simulink model "AcasXuClosedLoop_m_1_comp2017b"  (Simulink 9.0)
settings: Solver=VariableStepAuto  StartTime=0.0  StopTime=35  MaxStep=0.2  RelTol=1e-3
libraries: neural x1560, simulink x3
(part 5 of 60)

== system AcasXuClosedLoop_m_1_comp2017b/networks/AcasXu_5_1/Layer 3 ==
  "a{2} " : Inport  PortDimensions=50
      mask Display: e = 0.06;c1 = [120 230 180]/255;c2 = ([120 230 180]+0)/(256+128);c3 = ([120 230 180]+128)/(256+128);patch([0 1 1 0],[0 0 1 1],c1);patch([0 1 1 1-e 1-e 0+e],[0 0 1 1-e 0+e 0+e],c2);patch([0 0 1 1-e 0+e 0+e],[0 1 1 1-e 1-e 0+e],c3);plot([0 1 1 0 0],[0 0 1 1 0]);
  Delays 1 : Subsystem
      mask Display: e = 0.06;c1 = [120 150 230]/255;c2 = ([120 150 230]+0)/383;c3 = ([120 150 230]+128)/383;patch([0 1 1 0],[0 0 1 1],c1);patch([0 1 1 1-e 1-e 0+e],[0 0 1 1-e 0+e 0+e],c2);patch([0 0 1 1-e 0+e 0+e],[0 1 1 1-e 1-e 0+e],c3);plot([0 1 1 0 0],[0 0 1 1 0]);disp('0');
  "LW{3,2}" : Subsystem
      mask Display: e = 0.06;c1 = [120 150 230]/255;c2 = ([120 150 230]+0)/383;c3 = ([120 150 230]+128)/383;patch([0 1 1 0],[0 0 1 1],c1);patch([0 1 1 1-e 1-e 0+e],[0 0 1 1-e 0+e 0+e],c2);patch([0 0 1 1-e 0+e 0+e],[0 1 1 1-e 1-e 0+e],c3);plot([0 1 1 0 0],[0 0 1 1 0]);disp('W');
  "b{3}" : Constant  Value=[0.272230000000000027515767442309879697859287261962890625;-1.0544800000000000839150970932678319513797760009765625;1.5729699999999999793232063893810845911502838134765625;1.4368700000000000915889586394769139587879180908203125;-0.422177999999999997715605104531277902424335479736328125;-0.98838400000000004030908939967048354446887969970703125;-0.021854800000000000614885919958396698348224163055419921875;-0.293798999999999976839859527899534441530704498291015625;1.0949400000000000243716158365714363753795623779296875;-0.0307898999999999985199838903326963190920650959014892578125;-0.06296770000000000144790845979514415375888347625732421875;-0.84011599999999997390176531553152017295360565185546875;0.503924000000000038568259697058238089084625244140625;0.2310029999999999861248767274446436204016208648681640625;-0.0287351999999999989376942011176652158610522747039794921875;1.1389400000000000634514663033769465982913970947265625;0.73577899999999996083488440490327775478363037109375;0.110875000000000001332267629550187848508358001708984375;-1.0117599999999999926814098216709680855274200439453125;-0.1491240000000000065494276668687234632670879364013671875;-1.0125100000000000211031192520749755203723907470703125;-1.1087100000000000843414227347238920629024505615234375;1.4243699999999999139532746994518674910068511962890625;0.298072999999999976861175809972337447106838226318359375;0.34876600000000002044231450781808234751224517822265625;0.277681000000000011151968237754772417247295379638671875;-1.259819999999999939888084554695524275302886962890625;-0.26194499999999998340882712000166065990924835205078125;0.14417299999999999560174046564497984945774078369140625;-0.29806100000000002037126023424207232892513275146484375;-1.0689100000000000267874611381557770073413848876953125;-0.0578330999999999983973708594930940307676792144775390625;0.600875999999999965694996717502363026142120361328125;0.61764699999999994606270092845079489052295684814453125;0.65339300000000000157029944602982141077518463134765625;-2.78967000000000009407585821463726460933685302734375;0.57990299999999994629007460389402695000171661376953125;0.480266000000000026215474235868896357715129852294921875;0.343100000000000016076029396572266705334186553955078125;-0.01002399999999999992417176741810180828906595706939697265625;-0.26819300000000001471534005759167484939098358154296875;-0.0729852000000000000756728013584506697952747344970703125;-0.0917779999999999984705567612763843499124050140380859375;0.446454999999999990745180866724695079028606414794921875;0.565514999999999989910293152206577360630035400390625;-0.54441200000000000702726765666739083826541900634765625;0.2388879999999999892423829805920831859111785888671875;0.0464861000000000024190427438952610827982425689697265625;0.55525100000000005007194658901426009833812713623046875;0.09514330000000000009396927680427324958145618438720703125]
      mask Display: e = 0.06;c1 = [120 150 230]/255;c2 = ([120 150 230]+0)/383;c3 = ([120 150 230]+128)/383;patch([0 1 1 0],[0 0 1 1],c1);patch([0 1 1 1-e 1-e 0+e],[0 0 1 1-e 0+e 0+e],c2);patch([0 0 1 1-e 0+e 0+e],[0 1 1 1-e 1-e 0+e],c3);plot([0 1 1 0 0],[0 0 1 1 0]);disp('b');
  netsum : Sum  Inputs=2  SaturateOnIntegerOverflow=on  InputSameDT=on  OutDataTypeStr=Inherit: Same as first input
      mask Display: e = 0.06;c1 = [120 150 230]/255;c2 = ([120 150 230]+0)/383;c3 = ([120 150 230]+128)/383;patch([0 1 1 0],[0 0 1 1],c1);patch([0 1 1 1-e 1-e 0+e],[0 0 1 1-e 0+e 0+e],c2);patch([0 0 1 1-e 0+e 0+e],[0 1 1 1-e 1-e 0+e],c3);plot([0 1 1 0 0],[0 0 1 1 0]);disp('+');
  poslin : neural/Transfer Functions/poslin
      settings: SourceProductName=Deep Learning Toolbox  SourceProductBaseCode=NN
  "a{3}" : Outport  InitialOutput=[0;0;0;0;0;0;0;0;0;0;0;0;0;0;0;0;0;0;0;0;0;0;0;0;0;0;0;0;0;0;0;0;0;0;0;0;0;0;0;0;0;0;0;0;0;0;0;0;0;0]
      mask Display: e = 0.06;c1 = [120 230 180]/255;c2 = ([120 230 180]+0)/(256+128);c3 = ([120 230 180]+128)/(256+128);patch([0 1 1 0],[0 0 1 1],c1);patch([0 1 1 1-e 1-e 0+e],[0 0 1 1-e 0+e 0+e],c2);patch([0 0 1 1-e 0+e 0+e],[0 1 1 1-e 1-e 0+e],c3);plot([0 1 1 0 0],[0 0 1 1 0]);
  wires:
  poslin/1 -> "a{3}"/1
  netsum/1 -> poslin/1
  "b{3}"/1 -> netsum/2
  "LW{3,2}"/1("lz{3,2}") -> netsum/1
  Delays 1/1("pd{3,2}") -> "LW{3,2}"/1("ad{3,2}")
  "a{2} "/1 -> Delays 1/1("p{3}")

== system AcasXuClosedLoop_m_1_comp2017b/networks/AcasXu_5_1/Layer 4 ==
  "a{3} " : Inport  PortDimensions=50
      mask Display: e = 0.06;c1 = [120 230 180]/255;c2 = ([120 230 180]+0)/(256+128);c3 = ([120 230 180]+128)/(256+128);patch([0 1 1 0],[0 0 1 1],c1);patch([0 1 1 1-e 1-e 0+e],[0 0 1 1-e 0+e 0+e],c2);patch([0 0 1 1-e 0+e 0+e],[0 1 1 1-e 1-e 0+e],c3);plot([0 1 1 0 0],[0 0 1 1 0]);
  Delays 1 : Subsystem
      mask Display: e = 0.06;c1 = [120 150 230]/255;c2 = ([120 150 230]+0)/383;c3 = ([120 150 230]+128)/383;patch([0 1 1 0],[0 0 1 1],c1);patch([0 1 1 1-e 1-e 0+e],[0 0 1 1-e 0+e 0+e],c2);patch([0 0 1 1-e 0+e 0+e],[0 1 1 1-e 1-e 0+e],c3);plot([0 1 1 0 0],[0 0 1 1 0]);disp('0');
  "LW{4,3}" : Subsystem
      mask Display: e = 0.06;c1 = [120 150 230]/255;c2 = ([120 150 230]+0)/383;c3 = ([120 150 230]+128)/383;patch([0 1 1 0],[0 0 1 1],c1);patch([0 1 1 1-e 1-e 0+e],[0 0 1 1-e 0+e 0+e],c2);patch([0 0 1 1-e 0+e 0+e],[0 1 1 1-e 1-e 0+e],c3);plot([0 1 1 0 0],[0 0 1 1 0]);disp('W');
  "b{4}" : Constant  Value=[-0.56321100000000001717381792332162149250507354736328125;-0.08371520000000000349960060930243344046175479888916015625;-1.1247599999999999820232687852694652974605560302734375;-2.294610000000000038511416278197430074214935302734375;0.94715000000000004742872761198668740689754486083984375;0.6754759999999999653397253496223129332065582275390625;-0.0302282000000000002082334304986943607218563556671142578125;-0.0271720000000000017070789226636406965553760528564453125;0.0749916999999999944748196867294609546661376953125;0.269618999999999997552180275306454859673976898193359375;-1.1817299999999999471356204594485461711883544921875;-0.4554139999999999854907173357787542045116424560546875;0.446923000000000014697576489197672344744205474853515625;-0.057709799999999998598720907239112420938909053802490234375;1.61118000000000005655920176650397479534149169921875;-2.418950000000000155608859131461940705776214599609375;-1.77967999999999992866150932968594133853912353515625;1.2901100000000000900257646208046935498714447021484375;0.360455000000000025384139235029579140245914459228515625;-0.78738200000000002631850293255411088466644287109375;0.1540680000000000104964925640160799957811832427978515625;1.3485199999999999409538986583356745541095733642578125;0.429234999999999977671194528738851658999919891357421875;-0.9072050000000000391509047403815202414989471435546875;-0.0237161000000000003751221555603478918783366680145263671875;0.345032999999999978602005512584582902491092681884765625;-0.185626000000000013212542171459062956273555755615234375;-1.07753999999999994230392985627986490726470947265625;0.958077999999999985192289386759512126445770263671875;-0.1085939999999999960866858828012482263147830963134765625;0.70110499999999997822186514895292930305004119873046875;-0.04197879999999999667270600411939085461199283599853515625;0.90369500000000002604139126560767181217670440673828125;0.1236130000000000006554756737386924214661121368408203125;-2.5700599999999997891109160264022648334503173828125;0.1852290000000000047553072590744704939424991607666015625;0.74212599999999995237232042200048454105854034423828125;0.53999699999999994926014323937124572694301605224609375;-0.354986999999999996990851514055975712835788726806640625;1.3218799999999999439381781485280953347682952880859375;-1.9547000000000001040945107888546772301197052001953125;0.74946100000000004381917051432537846267223358154296875;-0.34008300000000002416555844320100732147693634033203125;-0.01237429999999999953586016232520705671049654483795166015625;-2.613170000000000214868123293854296207427978515625;-0.268365000000000020197177263980847783386707305908203125;-0.743109000000000019525714378687553107738494873046875;-2.42053999999999991388222042587585747241973876953125;-0.85676300000000005230305077930097468197345733642578125;-0.75692999999999999172217712839483283460140228271484375]
      mask Display: e = 0.06;c1 = [120 150 230]/255;c2 = ([120 150 230]+0)/383;c3 = ([120 150 230]+128)/383;patch([0 1 1 0],[0 0 1 1],c1);patch([0 1 1 1-e 1-e 0+e],[0 0 1 1-e 0+e 0+e],c2);patch([0 0 1 1-e 0+e 0+e],[0 1 1 1-e 1-e 0+e],c3);plot([0 1 1 0 0],[0 0 1 1 0]);disp('b');
  netsum : Sum  Inputs=2  SaturateOnIntegerOverflow=on  InputSameDT=on  OutDataTypeStr=Inherit: Same as first input
      mask Display: e = 0.06;c1 = [120 150 230]/255;c2 = ([120 150 230]+0)/383;c3 = ([120 150 230]+128)/383;patch([0 1 1 0],[0 0 1 1],c1);patch([0 1 1 1-e 1-e 0+e],[0 0 1 1-e 0+e 0+e],c2);patch([0 0 1 1-e 0+e 0+e],[0 1 1 1-e 1-e 0+e],c3);plot([0 1 1 0 0],[0 0 1 1 0]);disp('+');
  poslin : neural/Transfer Functions/poslin
      settings: SourceProductName=Deep Learning Toolbox  SourceProductBaseCode=NN
  "a{4}" : Outport  InitialOutput=[0;0;0;0;0;0;0;0;0;0;0;0;0;0;0;0;0;0;0;0;0;0;0;0;0;0;0;0;0;0;0;0;0;0;0;0;0;0;0;0;0;0;0;0;0;0;0;0;0;0]
      mask Display: e = 0.06;c1 = [120 230 180]/255;c2 = ([120 230 180]+0)/(256+128);c3 = ([120 230 180]+128)/(256+128);patch([0 1 1 0],[0 0 1 1],c1);patch([0 1 1 1-e 1-e 0+e],[0 0 1 1-e 0+e 0+e],c2);patch([0 0 1 1-e 0+e 0+e],[0 1 1 1-e 1-e 0+e],c3);plot([0 1 1 0 0],[0 0 1 1 0]);
  wires:
  poslin/1 -> "a{4}"/1
  netsum/1 -> poslin/1
  "b{4}"/1 -> netsum/2
  "LW{4,3}"/1("lz{4,3}") -> netsum/1
  Delays 1/1("pd{4,3}") -> "LW{4,3}"/1("ad{4,3}")
  "a{3} "/1 -> Delays 1/1("p{4}")

== system AcasXuClosedLoop_m_1_comp2017b/networks/AcasXu_5_1/Layer 5 ==
  "a{4} " : Inport  PortDimensions=50
      mask Display: e = 0.06;c1 = [120 230 180]/255;c2 = ([120 230 180]+0)/(256+128);c3 = ([120 230 180]+128)/(256+128);patch([0 1 1 0],[0 0 1 1],c1);patch([0 1 1 1-e 1-e 0+e],[0 0 1 1-e 0+e 0+e],c2);patch([0 0 1 1-e 0+e 0+e],[0 1 1 1-e 1-e 0+e],c3);plot([0 1 1 0 0],[0 0 1 1 0]);
  Delays 1 : Subsystem
      mask Display: e = 0.06;c1 = [120 150 230]/255;c2 = ([120 150 230]+0)/383;c3 = ([120 150 230]+128)/383;patch([0 1 1 0],[0 0 1 1],c1);patch([0 1 1 1-e 1-e 0+e],[0 0 1 1-e 0+e 0+e],c2);patch([0 0 1 1-e 0+e 0+e],[0 1 1 1-e 1-e 0+e],c3);plot([0 1 1 0 0],[0 0 1 1 0]);disp('0');
  "LW{5,4}" : Subsystem
      mask Display: e = 0.06;c1 = [120 150 230]/255;c2 = ([120 150 230]+0)/383;c3 = ([120 150 230]+128)/383;patch([0 1 1 0],[0 0 1 1],c1);patch([0 1 1 1-e 1-e 0+e],[0 0 1 1-e 0+e 0+e],c2);patch([0 0 1 1-e 0+e 0+e],[0 1 1 1-e 1-e 0+e],c3);plot([0 1 1 0 0],[0 0 1 1 0]);disp('W');
  "b{5}" : Constant  Value=[-0.14140900000000000691358081894577480852603912353515625;0.1905590000000000061586291622006683610379695892333984375;-0.415742000000000000436983782492461614310741424560546875;-0.4857460000000000110986775325727649033069610595703125;-0.63958999999999999186428567554685287177562713623046875;0.1415129999999999999005240169935859739780426025390625;-0.9334029999999999827053898115991614758968353271484375;-0.506637000000000004007461029686965048313140869140625;-0.26172099999999998143351831458858214318752288818359375;0.393040000000000000479616346638067625463008880615234375;-0.00924582000000000008010925256485279533080756664276123046875;-0.1550370000000000081374906812925473786890506744384765625;-0.2291229999999999933368854954096605069935321807861328125;0.08985390000000000043645087544064153917133808135986328125;0.188946000000000002838618229361600242555141448974609375;0.14731199999999999850075482754618860781192779541015625;-0.1534600000000000130651045537888421677052974700927734375;-0.74877099999999996438049265634617768228054046630859375;-0.47552600000000000424194013248779810965061187744140625;0.4521399999999999863575794734060764312744140625;0.53029099999999995684873965728911571204662322998046875;-0.024597700000000000120081722343456931412220001220703125;1.357720000000000037942982089589349925518035888671875;0.537077000000000026602720026858150959014892578125;0.1478450000000000041922021409845910966396331787109375;-1.1116800000000000014921397450962103903293609619140625;0.90564400000000000456878979093744419515132904052734375;0.37051000000000000600408611717284657061100006103515625;-0.72400600000000003841904572254861705005168914794921875;-0.568551999999999946311390885966829955577850341796875;0.298362999999999989331200822562095709145069122314453125;-0.1264849999999999863309341208150726743042469024658203125;-0.41675899999999999057109789646347053349018096923828125;-0.369709000000000009844569603956188075244426727294921875;-1.809250000000000024868995751603506505489349365234375;-2.147089999999999943014472592039965093135833740234375;0.49527799999999999602806610710103996098041534423828125;-0.05108780000000000265369948238003416918218135833740234375;-0.75050399999999994893329358092159964144229888916015625;0.385384000000000004337863401815411634743213653564453125;-0.1876790000000000124824595104655600152909755706787109375;-0.762844999999999995310417943983338773250579833984375;-0.264797000000000004593658786689047701656818389892578125;-2.487999999999999989341858963598497211933135986328125;-0.019833599999999999841104880715647595934569835662841796875;-0.540321000000000051244342103018425405025482177734375;-0.1260499999999999953814722175593487918376922607421875;-0.1066560000000000008046896482483134604990482330322265625;0.460942000000000018378187860434991307556629180908203125;-1.1129800000000000803623834144673310220241546630859375]
      mask Display: e = 0.06;c1 = [120 150 230]/255;c2 = ([120 150 230]+0)/383;c3 = ([120 150 230]+128)/383;patch([0 1 1 0],[0 0 1 1],c1);patch([0 1 1 1-e 1-e 0+e],[0 0 1 1-e 0+e 0+e],c2);patch([0 0 1 1-e 0+e 0+e],[0 1 1 1-e 1-e 0+e],c3);plot([0 1 1 0 0],[0 0 1 1 0]);disp('b');
  netsum : Sum  Inputs=2  SaturateOnIntegerOverflow=on  InputSameDT=on  OutDataTypeStr=Inherit: Same as first input
      mask Display: e = 0.06;c1 = [120 150 230]/255;c2 = ([120 150 230]+0)/383;c3 = ([120 150 230]+128)/383;patch([0 1 1 0],[0 0 1 1],c1);patch([0 1 1 1-e 1-e 0+e],[0 0 1 1-e 0+e 0+e],c2);patch([0 0 1 1-e 0+e 0+e],[0 1 1 1-e 1-e 0+e],c3);plot([0 1 1 0 0],[0 0 1 1 0]);disp('+');
  poslin : neural/Transfer Functions/poslin
      settings: SourceProductName=Deep Learning Toolbox  SourceProductBaseCode=NN
  "a{5}" : Outport  InitialOutput=[0;0;0;0;0;0;0;0;0;0;0;0;0;0;0;0;0;0;0;0;0;0;0;0;0;0;0;0;0;0;0;0;0;0;0;0;0;0;0;0;0;0;0;0;0;0;0;0;0;0]
      mask Display: e = 0.06;c1 = [120 230 180]/255;c2 = ([120 230 180]+0)/(256+128);c3 = ([120 230 180]+128)/(256+128);patch([0 1 1 0],[0 0 1 1],c1);patch([0 1 1 1-e 1-e 0+e],[0 0 1 1-e 0+e 0+e],c2);patch([0 0 1 1-e 0+e 0+e],[0 1 1 1-e 1-e 0+e],c3);plot([0 1 1 0 0],[0 0 1 1 0]);
  wires:
  poslin/1 -> "a{5}"/1
  netsum/1 -> poslin/1
  "b{5}"/1 -> netsum/2
  "LW{5,4}"/1("lz{5,4}") -> netsum/1
  Delays 1/1("pd{5,4}") -> "LW{5,4}"/1("ad{5,4}")
  "a{4} "/1 -> Delays 1/1("p{5}")

== system AcasXuClosedLoop_m_1_comp2017b/networks/AcasXu_5_1/Layer 6 ==
  "a{5} " : Inport  PortDimensions=50
      mask Display: e = 0.06;c1 = [120 230 180]/255;c2 = ([120 230 180]+0)/(256+128);c3 = ([120 230 180]+128)/(256+128);patch([0 1 1 0],[0 0 1 1],c1);patch([0 1 1 1-e 1-e 0+e],[0 0 1 1-e 0+e 0+e],c2);patch([0 0 1 1-e 0+e 0+e],[0 1 1 1-e 1-e 0+e],c3);plot([0 1 1 0 0],[0 0 1 1 0]);
  Delays 1 : Subsystem
      mask Display: e = 0.06;c1 = [120 150 230]/255;c2 = ([120 150 230]+0)/383;c3 = ([120 150 230]+128)/383;patch([0 1 1 0],[0 0 1 1],c1);patch([0 1 1 1-e 1-e 0+e],[0 0 1 1-e 0+e 0+e],c2);patch([0 0 1 1-e 0+e 0+e],[0 1 1 1-e 1-e 0+e],c3);plot([0 1 1 0 0],[0 0 1 1 0]);disp('0');
  "LW{6,5}" : Subsystem
      mask Display: e = 0.06;c1 = [120 150 230]/255;c2 = ([120 150 230]+0)/383;c3 = ([120 150 230]+128)/383;patch([0 1 1 0],[0 0 1 1],c1);patch([0 1 1 1-e 1-e 0+e],[0 0 1 1-e 0+e 0+e],c2);patch([0 0 1 1-e 0+e 0+e],[0 1 1 1-e 1-e 0+e],c3);plot([0 1 1 0 0],[0 0 1 1 0]);disp('W');
  "b{6}" : Constant  Value=[-0.000797148000000000018887280628376856839167885482311248779296875;-1.13483000000000000540012479177676141262054443359375;-0.014565200000000000368771679859491996467113494873046875;-0.070781700000000002948041810668655671179294586181640625;-0.8549830000000000485016471429844386875629425048828125;0.046092000000000001025401985543794580735266208648681640625;-0.391602999999999978886222606888622976839542388916015625;-0.2224299999999999888355972643694258294999599456787109375;-0.0355173000000000016473933328597922809422016143798828125;-0.052159299999999998720401350738029577769339084625244140625;-0.06426869999999999805151418286186526529490947723388671875;0.305495000000000016537882174816331826150417327880859375;-0.4729900000000000215294448935310356318950653076171875;0.2437350000000000072031269837680156342685222625732421875;0.1107619999999999993445243262613075785338878631591796875;-0.224841000000000013070433624307042919099330902099609375;-2.267920000000000158024704433046281337738037109375;-0.61285800000000001386268877467955462634563446044921875;0.1929200000000000081445961086501483805477619171142578125;-0.10164900000000000324096305348575697280466556549072265625;-0.38281500000000001637801005927030928432941436767578125;-0.442025999999999974487963072533602826297283172607421875;-1.0189399999999999568700559393619187176227569580078125;-1.2009600000000000274980038739158771932125091552734375;-1.096880000000000077164941103546880185604095458984375;-0.8883630000000000137561073643155395984649658203125;-0.06573969999999999813677931115307728759944438934326171875;0.045780599999999997684607677683743531815707683563232421875;-0.14674799999999998956212721168412826955318450927734375;-0.0873474999999999945909934240262373350560665130615234375;0.0669754999999999933724126321976655162870883941650390625;-0.07503859999999999697006813903499278239905834197998046875;0.0223789999999999995872190794443667982704937458038330078125;-0.323334999999999983533172098759678192436695098876953125;-0.24221599999999998686917024315334856510162353515625;-0.54533100000000001017497197608463466167449951171875;0.013239700000000000079669604247101233340799808502197265625;0.13325500000000001232791646543773822486400604248046875;0.0958935999999999955090146386282867752015590667724609375;-0.2077440000000000119850795954334898851811885833740234375;-0.1618380000000000096260777127099572680890560150146484375;-0.78143499999999999072741729833069257438182830810546875;-0.44243500000000002270184040753520093858242034912109375;0.1299849999999999894395585897655109874904155731201171875;-0.200556000000000012040146657454897649586200714111328125;0.022201999999999999513278226004331372678279876708984375;-1.096060000000000034248159863636828958988189697265625;0.12263100000000000389110965670624864287674427032470703125;0.03959859999999999768949265899209422059357166290283203125;0.153768999999999989025667446185252629220485687255859375]
      mask Display: e = 0.06;c1 = [120 150 230]/255;c2 = ([120 150 230]+0)/383;c3 = ([120 150 230]+128)/383;patch([0 1 1 0],[0 0 1 1],c1);patch([0 1 1 1-e 1-e 0+e],[0 0 1 1-e 0+e 0+e],c2);patch([0 0 1 1-e 0+e 0+e],[0 1 1 1-e 1-e 0+e],c3);plot([0 1 1 0 0],[0 0 1 1 0]);disp('b');
  netsum : Sum  Inputs=2  SaturateOnIntegerOverflow=on  InputSameDT=on  OutDataTypeStr=Inherit: Same as first input
      mask Display: e = 0.06;c1 = [120 150 230]/255;c2 = ([120 150 230]+0)/383;c3 = ([120 150 230]+128)/383;patch([0 1 1 0],[0 0 1 1],c1);patch([0 1 1 1-e 1-e 0+e],[0 0 1 1-e 0+e 0+e],c2);patch([0 0 1 1-e 0+e 0+e],[0 1 1 1-e 1-e 0+e],c3);plot([0 1 1 0 0],[0 0 1 1 0]);disp('+');
  poslin : neural/Transfer Functions/poslin
      settings: SourceProductName=Deep Learning Toolbox  SourceProductBaseCode=NN
  "a{6}" : Outport  InitialOutput=[0;0;0;0;0;0;0;0;0;0;0;0;0;0;0;0;0;0;0;0;0;0;0;0;0;0;0;0;0;0;0;0;0;0;0;0;0;0;0;0;0;0;0;0;0;0;0;0;0;0]
      mask Display: e = 0.06;c1 = [120 230 180]/255;c2 = ([120 230 180]+0)/(256+128);c3 = ([120 230 180]+128)/(256+128);patch([0 1 1 0],[0 0 1 1],c1);patch([0 1 1 1-e 1-e 0+e],[0 0 1 1-e 0+e 0+e],c2);patch([0 0 1 1-e 0+e 0+e],[0 1 1 1-e 1-e 0+e],c3);plot([0 1 1 0 0],[0 0 1 1 0]);
  wires:
  poslin/1 -> "a{6}"/1
  netsum/1 -> poslin/1
  "b{6}"/1 -> netsum/2
  "LW{6,5}"/1("lz{6,5}") -> netsum/1
  Delays 1/1("pd{6,5}") -> "LW{6,5}"/1("ad{6,5}")
  "a{5} "/1 -> Delays 1/1("p{6}")

== system AcasXuClosedLoop_m_1_comp2017b/networks/AcasXu_5_1/Layer 7 ==
  "a{6} " : Inport  PortDimensions=50
      mask Display: e = 0.06;c1 = [120 230 180]/255;c2 = ([120 230 180]+0)/(256+128);c3 = ([120 230 180]+128)/(256+128);patch([0 1 1 0],[0 0 1 1],c1);patch([0 1 1 1-e 1-e 0+e],[0 0 1 1-e 0+e 0+e],c2);patch([0 0 1 1-e 0+e 0+e],[0 1 1 1-e 1-e 0+e],c3);plot([0 1 1 0 0],[0 0 1 1 0]);
  Delays 1 : Subsystem
      mask Display: e = 0.06;c1 = [120 150 230]/255;c2 = ([120 150 230]+0)/383;c3 = ([120 150 230]+128)/383;patch([0 1 1 0],[0 0 1 1],c1);patch([0 1 1 1-e 1-e 0+e],[0 0 1 1-e 0+e 0+e],c2);patch([0 0 1 1-e 0+e 0+e],[0 1 1 1-e 1-e 0+e],c3);plot([0 1 1 0 0],[0 0 1 1 0]);disp('0');
  "LW{7,6}" : Subsystem
      mask Display: e = 0.06;c1 = [120 150 230]/255;c2 = ([120 150 230]+0)/383;c3 = ([120 150 230]+128)/383;patch([0 1 1 0],[0 0 1 1],c1);patch([0 1 1 1-e 1-e 0+e],[0 0 1 1-e 0+e 0+e],c2);patch([0 0 1 1-e 0+e 0+e],[0 1 1 1-e 1-e 0+e],c3);plot([0 1 1 0 0],[0 0 1 1 0]);disp('W');
  "b{7}" : Constant  Value=[-0.0203227999999999985047960393558241776190698146820068359375;0.018330699999999998384137001039562164805829524993896484375;-0.018534899999999999986588505862528108991682529449462890625;0.0181401999999999986867837975523798377253115177154541015625;-0.0183874000000000016596946039726390154100954532623291015625]
      mask Display: e = 0.06;c1 = [120 150 230]/255;c2 = ([120 150 230]+0)/383;c3 = ([120 150 230]+128)/383;patch([0 1 1 0],[0 0 1 1],c1);patch([0 1 1 1-e 1-e 0+e],[0 0 1 1-e 0+e 0+e],c2);patch([0 0 1 1-e 0+e 0+e],[0 1 1 1-e 1-e 0+e],c3);plot([0 1 1 0 0],[0 0 1 1 0]);disp('b');
  netsum : Sum  Inputs=2  SaturateOnIntegerOverflow=on  InputSameDT=on  OutDataTypeStr=Inherit: Same as first input
      mask Display: e = 0.06;c1 = [120 150 230]/255;c2 = ([120 150 230]+0)/383;c3 = ([120 150 230]+128)/383;patch([0 1 1 0],[0 0 1 1],c1);patch([0 1 1 1-e 1-e 0+e],[0 0 1 1-e 0+e 0+e],c2);patch([0 0 1 1-e 0+e 0+e],[0 1 1 1-e 1-e 0+e],c3);plot([0 1 1 0 0],[0 0 1 1 0]);disp('+');
  purelin : neural/Transfer Functions/purelin
      settings: SourceProductName=Deep Learning Toolbox
  "a{7}" : Outport  InitialOutput=[0;0;0;0;0]
      mask Display: e = 0.06;c1 = [120 230 180]/255;c2 = ([120 230 180]+0)/(256+128);c3 = ([120 230 180]+128)/(256+128);patch([0 1 1 0],[0 0 1 1],c1);patch([0 1 1 1-e 1-e 0+e],[0 0 1 1-e 0+e 0+e],c2);patch([0 0 1 1-e 0+e 0+e],[0 1 1 1-e 1-e 0+e],c3);plot([0 1 1 0 0],[0 0 1 1 0]);
  wires:
  purelin/1 -> "a{7}"/1
  netsum/1 -> purelin/1
  "b{7}"/1 -> netsum/2
  "LW{7,6}"/1("lz{7,6}") -> netsum/1
  Delays 1/1("pd{7,6}") -> "LW{7,6}"/1("ad{7,6}")
  "a{6} "/1 -> Delays 1/1("p{7}")

== system AcasXuClosedLoop_m_1_comp2017b/networks/AcasXu_5_1/Process Input 1 ==
  x : Inport  PortDimensions=5
      mask Display: e = 0.06;c1 = [120 230 180]/255;c2 = ([120 230 180]+0)/(256+128);c3 = ([120 230 180]+128)/(256+128);patch([0 1 1 0],[0 0 1 1],c1);patch([0 1 1 1-e 1-e 0+e],[0 0 1 1-e 0+e 0+e],c2);patch([0 0 1 1-e 0+e 0+e],[0 1 1 1-e 1-e 0+e],c3);plot([0 1 1 0 0],[0 0 1 1 0]);
  p : Outport  PortDimensions=5
      mask Display: e = 0.06;c1 = [120 230 180]/255;c2 = ([120 230 180]+0)/(256+128);c3 = ([120 230 180]+128)/(256+128);patch([0 1 1 0],[0 0 1 1],c1);patch([0 1 1 1-e 1-e 0+e],[0 0 1 1-e 0+e 0+e],c2);patch([0 0 1 1-e 0+e 0+e],[0 1 1 1-e 1-e 0+e],c3);plot([0 1 1 0 0],[0 0 1 1 0]);
  wires:
  x/1 -> p/1

== system AcasXuClosedLoop_m_1_comp2017b/networks/AcasXu_5_1/Process Output 1 ==
  a : Inport  PortDimensions=5
      mask Display: e = 0.06;c1 = [120 230 180]/255;c2 = ([120 230 180]+0)/(256+128);c3 = ([120 230 180]+128)/(256+128);patch([0 1 1 0],[0 0 1 1],c1);patch([0 1 1 1-e 1-e 0+e],[0 0 1 1-e 0+e 0+e],c2);patch([0 0 1 1-e 0+e 0+e],[0 1 1 1-e 1-e 0+e],c3);plot([0 1 1 0 0],[0 0 1 1 0]);
  y : Outport  PortDimensions=5
      mask Display: e = 0.06;c1 = [120 230 180]/255;c2 = ([120 230 180]+0)/(256+128);c3 = ([120 230 180]+128)/(256+128);patch([0 1 1 0],[0 0 1 1],c1);patch([0 1 1 1-e 1-e 0+e],[0 0 1 1-e 0+e 0+e],c2);patch([0 0 1 1-e 0+e 0+e],[0 1 1 1-e 1-e 0+e],c3);plot([0 1 1 0 0],[0 0 1 1 0]);
  wires:
  a/1 -> y/1

== system AcasXuClosedLoop_m_1_comp2017b/networks/AcasXu_1_1/Layer 1/Delays 1 ==
  "p{1}" : Inport  PortDimensions=5
      mask Display: e = 0.06;c1 = [120 230 180]/255;c2 = ([120 230 180]+0)/(256+128);c3 = ([120 230 180]+128)/(256+128);patch([0 1 1 0],[0 0 1 1],c1);patch([0 1 1 1-e 1-e 0+e],[0 0 1 1-e 0+e 0+e],c2);patch([0 0 1 1-e 0+e 0+e],[0 1 1 1-e 1-e 0+e],c3);plot([0 1 1 0 0],[0 0 1 1 0]);
  mux : Mux  Inputs=1
      mask Display: e = 0.06;c1 = [120 150 230]/255;c2 = ([120 150 230]+0)/383;c3 = ([120 150 230]+128)/383;patch([0 1 1 0],[0 0 1 1],c1);patch([0 1 1 1-e 1-e 0+e],[0 0 1 1-e 0+e 0+e],c2);patch([0 0 1 1-e 0+e 0+e],[0 1 1 1-e 1-e 0+e],c3);plot([0 1 1 0 0],[0 0 1 1 0]);disp('Mux')
  "pd{1,1}" : Outport
      mask Display: e = 0.06;c1 = [120 230 180]/255;c2 = ([120 230 180]+0)/(256+128);c3 = ([120 230 180]+128)/(256+128);patch([0 1 1 0],[0 0 1 1],c1);patch([0 1 1 1-e 1-e 0+e],[0 0 1 1-e 0+e 0+e],c2);patch([0 0 1 1-e 0+e 0+e],[0 1 1 1-e 1-e 0+e],c3);plot([0 1 1 0 0],[0 0 1 1 0]);
  wires:
  mux/1 -> "pd{1,1}"/1
  "p{1}"/1 -> mux/1
